simulink model "ClimaCenCfgMgr"  (R2022b)
settings: Solver=FixedStepDiscrete  StartTime=0.0  StopTime=10.0  FixedStep=0.1  RelTol=1e-3
(part 2 of 2)

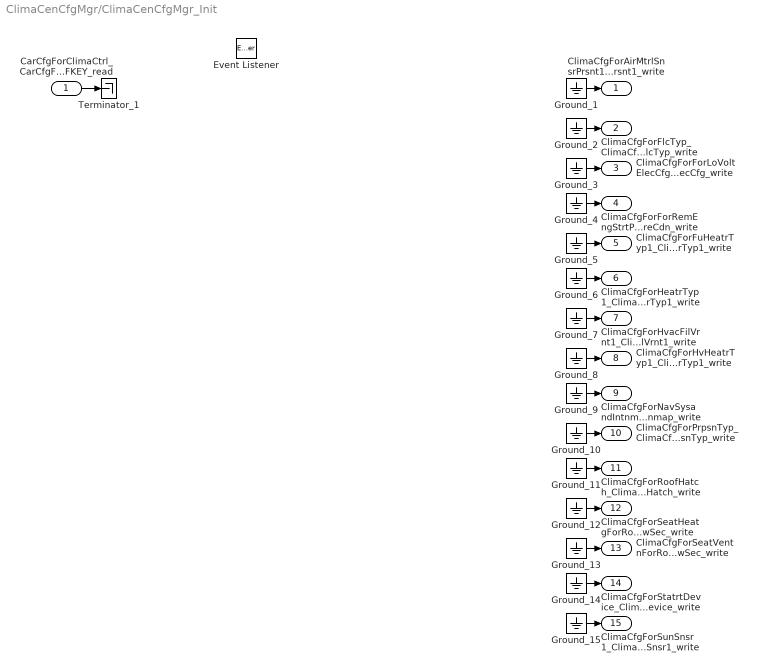
== system ClimaCenCfgMgr/ClimaCenCfgMgr_Init ==
  CarCfgForClimaCtrl_CarCfgForRFKEY_read : Inport
  Event Listener : EventListener  EventName=reset
  Ground_{1..15} (15 alike) : Ground
  Terminator_1 : Terminator
  ClimaCfgForAirMtrlSnsrPrsnt1_ClimaCfgForAirMtrlSnsrPrsnt1_write : Outport
  ClimaCfgForFlcTyp_ClimaCfgForFlcTyp_write : Outport  Port=2
  ClimaCfgForForLoVoltElecCfg_ClimaCfgForForLoVoltElecCfg_write : Outport  Port=3
  ClimaCfgForForRemEngStrtPreCdn_ClimaCfgForForRemEngStrtPreCdn_write : Outport  Port=4
  ClimaCfgForFuHeatrTyp1_ClimaCfgForFuHeatrTyp1_write : Outport  Port=5
  ClimaCfgForHeatrTyp1_ClimaCfgForHeatrTyp1_write : Outport  Port=6
  ClimaCfgForHvacFilVrnt1_ClimaCfgForHvacFilVrnt1_write : Outport  Port=7
  ClimaCfgForHvHeatrTyp1_ClimaCfgForHvHeatrTyp1_write : Outport  Port=8
  ClimaCfgForNavSysandIntnmap_ClimaCfgForNavSysandIntnmap_write : Outport  Port=9
  ClimaCfgForPrpsnTyp_ClimaCfgForPrpsnTyp_write : Outport  Port=10
  ClimaCfgForRoofHatch_ClimaCfgForRoofHatch_write : Outport  Port=11
  ClimaCfgForSeatHeatgForRowSec_ClimaCfgForSeatHeatgForRowSec_write : Outport  Port=12
  ClimaCfgForSeatVentnForRowSec_ClimaCfgForSeatVentnForRowSec_write : Outport  Port=13
  ClimaCfgForStatrtDevice_ClimaCfgForStartDevice_write : Outport  Port=14
  ClimaCfgForSunSnsr1_ClimaCfgForSunSnsr1_write : Outport  Port=15
  wires:
  CarCfgForClimaCtrl_CarCfgForRFKEY_read/1 -> Terminator_1/1
  Ground_1/1 -> ClimaCfgForAirMtrlSnsrPrsnt1_ClimaCfgForAirMtrlSnsrPrsnt1_write/1
  Ground_2/1 -> ClimaCfgForFlcTyp_ClimaCfgForFlcTyp_write/1
  Ground_3/1 -> ClimaCfgForForLoVoltElecCfg_ClimaCfgForForLoVoltElecCfg_write/1
  Ground_4/1 -> ClimaCfgForForRemEngStrtPreCdn_ClimaCfgForForRemEngStrtPreCdn_write/1
  Ground_5/1 -> ClimaCfgForFuHeatrTyp1_ClimaCfgForFuHeatrTyp1_write/1
  Ground_6/1 -> ClimaCfgForHeatrTyp1_ClimaCfgForHeatrTyp1_write/1
  Ground_7/1 -> ClimaCfgForHvacFilVrnt1_ClimaCfgForHvacFilVrnt1_write/1
  Ground_8/1 -> ClimaCfgForHvHeatrTyp1_ClimaCfgForHvHeatrTyp1_write/1
  Ground_9/1 -> ClimaCfgForNavSysandIntnmap_ClimaCfgForNavSysandIntnmap_write/1
  Ground_10/1 -> ClimaCfgForPrpsnTyp_ClimaCfgForPrpsnTyp_write/1
  Ground_11/1 -> ClimaCfgForRoofHatch_ClimaCfgForRoofHatch_write/1
  Ground_12/1 -> ClimaCfgForSeatHeatgForRowSec_ClimaCfgForSeatHeatgForRowSec_write/1
  Ground_13/1 -> ClimaCfgForSeatVentnForRowSec_ClimaCfgForSeatVentnForRowSec_write/1
  Ground_14/1 -> ClimaCfgForStatrtDevice_ClimaCfgForStartDevice_write/1
  Ground_15/1 -> ClimaCfgForSunSnsr1_ClimaCfgForSunSnsr1_write/1

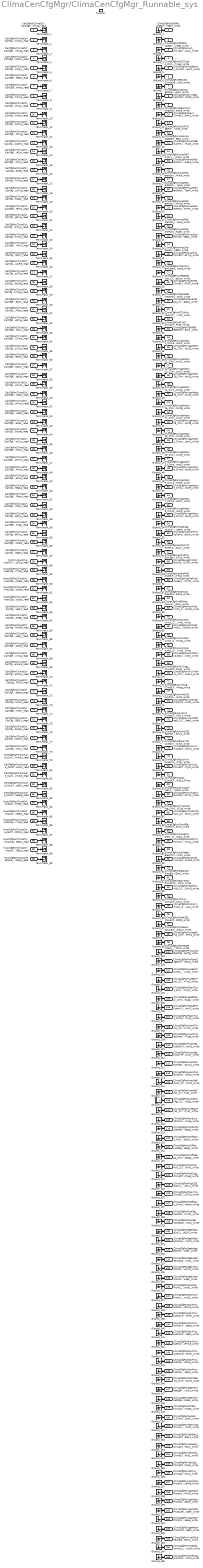
== system ClimaCenCfgMgr/ClimaCenCfgMgr_Runnable_sys ==
  CarCfgForClimaCtrl_CarCfgForAirClng_read : Inport
  CarCfgForClimaCtrl_CarCfgForAirClnrAdv_read : Inport  Port=2
  CarCfgForClimaCtrl_CarCfgForAirFrag_read : Inport  Port=3
  CarCfgForClimaCtrl_CarCfgForAirMtrlSnsr_read : Inport  Port=4
  CarCfgForClimaCtrl_CarCfgForAirQly_read : Inport  Port=5
  CarCfgForClimaCtrl_CarCfgForAmntOfSeat_read : Inport  Port=6
  CarCfgForClimaCtrl_CarCfgForAutDemst_read : Inport  Port=7
  CarCfgForClimaCtrl_CarCfgForAuxWtrPmp_read : Inport  Port=8
  CarCfgForClimaCtrl_CarCfgForBypVlvInHvac_read : Inport  Port=9
  CarCfgForClimaCtrl_CarCfgForClsVlvInHvac_read : Inport  Port=10
  CarCfgForClimaCtrl_CarCfgForCmprAcTyp_read : Inport  Port=11
  CarCfgForClimaCtrl_CarCfgForCmptmtClng_read : Inport  Port=12
  CarCfgForClimaCtrl_CarCfgForElecVentAdjmt_read : Inport  Port=13
  CarCfgForClimaCtrl_CarCfgForExtVentnClimaCtrl_read : Inport  Port=14
  CarCfgForClimaCtrl_CarCfgForFlcTyp_read : Inport  Port=15
  CarCfgForClimaCtrl_CarCfgForFoldCSD_read : Inport  Port=16
  CarCfgForClimaCtrl_CarCfgForFuTyp_read : Inport  Port=17
  CarCfgForClimaCtrl_CarCfgForGlassTyp_read : Inport  Port=18
  CarCfgForClimaCtrl_CarCfgForHeadRadar_read : Inport  Port=19
  CarCfgForClimaCtrl_CarCfgForHeatPmp_read : Inport  Port=20
  CarCfgForClimaCtrl_CarCfgForHeatrDurgDrvg_read : Inport  Port=21
  CarCfgForClimaCtrl_CarCfgForHeatrTyp_read : Inport  Port=22
  CarCfgForClimaCtrl_CarCfgForHvacFilTyp_read : Inport  Port=23
  CarCfgForClimaCtrl_CarCfgForHvacFlrSnsrForT_read : Inport  Port=24
  CarCfgForClimaCtrl_CarCfgForHvacHeatrSnsrT_read : Inport  Port=25
  CarCfgForClimaCtrl_CarCfgForHvacRe_read : Inport  Port=26
  CarCfgForClimaCtrl_CarCfgForHvacTyp_read : Inport  Port=27
  CarCfgForClimaCtrl_CarCfgForLoVoltElecCfg_read : Inport  Port=28
  CarCfgForClimaCtrl_CarCfgForNavSysandIntnmap_read : Inport  Port=29
  CarCfgForClimaCtrl_CarCfgForNrOfDoor_read : Inport  Port=30
  CarCfgForClimaCtrl_CarCfgForPipeInFuTank_read : Inport  Port=31
  CarCfgForClimaCtrl_CarCfgForPreClng_read : Inport  Port=32
  CarCfgForClimaCtrl_CarCfgForPrkgCDSNotAvl_read : Inport  Port=33
  CarCfgForClimaCtrl_CarCfgForPrkgClima_read : Inport  Port=34
  CarCfgForClimaCtrl_CarCfgForPrpsnTyp_read : Inport  Port=35
  CarCfgForClimaCtrl_CarCfgForRemEngStrtPreCdn_read : Inport  Port=36
  CarCfgForClimaCtrl_CarCfgForRoofHatch_read : Inport  Port=37
  CarCfgForClimaCtrl_CarCfgForSeatHeatgCal_read : Inport  Port=38
  CarCfgForClimaCtrl_CarCfgForSeatHeatgForRowFirst_read : Inport  Port=39
  CarCfgForClimaCtrl_CarCfgForSeatHeatgForRowSec_read : Inport  Port=40
  CarCfgForClimaCtrl_CarCfgForSeatVentnForRowFirst_read : Inport  Port=41
  CarCfgForClimaCtrl_CarCfgForSeatVentnForRowSec_read : Inport  Port=42
  CarCfgForClimaCtrl_CarCfgForSecRowVentnCenter_read : Inport  Port=43
  CarCfgForClimaCtrl_CarCfgForSecRowVentnSide_read : Inport  Port=44
  CarCfgForClimaCtrl_CarCfgForSmallFlowCyc_read : Inport  Port=45
  CarCfgForClimaCtrl_CarCfgForSmkSnsr_read : Inport  Port=46
  CarCfgForClimaCtrl_CarCfgForSnsrForCmptmtT_read : Inport  Port=47
  CarCfgForClimaCtrl_CarCfgForSnsrHumPrsnt_read : Inport  Port=48
  CarCfgForClimaCtrl_CarCfgForStartDevice_read : Inport  Port=49
  CarCfgForClimaCtrl_CarCfgForSteerWhlHeatg_read : Inport  Port=50
  CarCfgForClimaCtrl_CarCfgForSteerWhlPosn_read : Inport  Port=51
  CarCfgForClimaCtrl_CarCfgForSunSnsr_read : Inport  Port=52
  CarCfgForClimaCtrl_CarCfgForTelmNodTyp_read : Inport  Port=53
  CarCfgForClimaCtrl_CarCfgForThermMngt_read : Inport  Port=54
  CarCfgForClimaCtrl_CarCfgForVehTyp_read : Inport  Port=55
  CarCfgForClimaCtrl_CarCfgForVgaMotorExistSts_read : Inport  Port=56
  CarCfgForClimaCtrl_CarCfgForWindHeatd_read : Inport  Port=57
  CooltVlvOutpTyp_CooltVlvOutpTyp_read : Inport  Port=58
  HvacCfgForClimaCtrl_HvacCfgForHvacFrontBlowerType_read : Inport  Port=59
  HvacCfgForClimaCtrl_HvacCfgForHvacMiscExistSts_read : Inport  Port=60
  HvacCfgForClimaCtrl_HvacCfgForHvacMotorExistSts_read : Inport  Port=61
  HvacCfgForClimaCtrl_HvacCfgForHvacSensorExistSts_read : Inport  Port=62
  CarCfgForClimaCtrl_CarCfgFor3RDROWSEATHEAT_read : Inport  Port=63
  CarCfgForClimaCtrl_CarCfgFor3RDROWSEATVENTILATED_read : Inport  Port=64
  CarCfgForClimaCtrl_CarCfgForBRAND_read : Inport  Port=65
  CarCfgForClimaCtrl_CarCfgForCabinOxgenSupply_read : Inport  Port=66
  CarCfgForClimaCtrl_CarCfgForElectricLiftingModule_read : Inport  Port=67
  CarCfgForClimaCtrl_CarCfgForFloatingDriving_read : Inport  Port=68
  CarCfgForClimaCtrl_CarCfgForInteriorViewCamera_read : Inport  Port=69
  CarCfgForClimaCtrl_CarCfgForLifeDetection_read : Inport  Port=70
  CarCfgForClimaCtrl_CarCfgForMARKET_read : Inport  Port=71
  CarCfgForClimaCtrl_CarCfgForPowerSeats_read : Inport  Port=72
  CarCfgForClimaCtrl_CarCfgForPTCInFrontHVAC_read : Inport  Port=73
  CarCfgForClimaCtrl_CarCfgForRFKEY_read : Inport  Port=74
  CarCfgForClimaCtrl_CarCfgForTEMPEREDSTORAGEBOX_read : Inport  Port=75
  CarCfgForClimaCtrl_CarCfgForVentLiftMotorExiSts_read : Inport  Port=76
  CarCfgForClimaCtrl_CarCfgForWadingAssistSystem_read : Inport  Port=77
  CarCfgForClimaCtrl_CarCfgWindFollowHumanMov_read : Inport  Port=78
  CenCfgMgrForCompile_CarCfgForAirClnrAdv_read : Inport  Port=79
  CenCfgMgrForCompile_CarCfgForAutDemst_read : Inport  Port=80
  CenCfgMgrForCompile_CarCfgForCmprAcTyp3_read : Inport  Port=81
  CenCfgMgrForCompile_CarCfgForHeatrTypGly_read : Inport  Port=82
  CenCfgMgrForCompile_CarCfgForSteerWhlHeatg_read : Inport  Port=83
  HvacCfgForClimaCtrl_HvacCfgForAQSTypes_read : Inport  Port=84
  HvacCfgForClimaCtrl_HvacCfgForHvacLowSideRETXVESts_read : Inport  Port=85
  HvacCfgForClimaCtrl_HvacCfgForHvacReBlowerType_read : Inport  Port=86
  HvacCfgForClimaCtrl_HvacCfgForHvacReVentPstn_read : Inport  Port=87
  HvacCfgForClimaCtrl_HvacCfgForHvacSnsrRCmptmtESts_read : Inport  Port=88
  HvacCfgForClimaCtrl_HvacCfgForHvacSnsrReHexESts_read : Inport  Port=89
  HvacCfgReqVersionReplace_HvacCfgReqVersionReplace_read : Inport  Port=90
  function : TriggerPort  TriggerType=function-call  VariantControl=(inherit)  SampleTimeType=periodic  SampleTime=-1
  Ground_{1..115,117..165} (164 alike) : Ground
  Ground_116 : Subsystem
  Terminator_{1..90} (90 alike) : Terminator
  ClimaCfgFor3RDROWSEATHEAT_ClimaCfgFor3RDROWSEATHEAT_write : Outport
      settings: EnsureOutportIsVirtual=on
  ClimaCfgFor3RDROWSEATVENTILATED_ClimaCfgFor3RDROWSEATVENTILATED_write : Outport  Port=2
      settings: EnsureOutportIsVirtual=on
  ClimaCfgForAAC_ClimaCfgForAAC_write : Outport  Port=3
      settings: EnsureOutportIsVirtual=on
  ClimaCfgForAQSTypes_ClimaCfgForAQSTypes_write : Outport  Port=4
      settings: EnsureOutportIsVirtual=on
  ClimaCfgForAutDemst_ClimaCfgForAutDemst_write : Outport  Port=5
      settings: EnsureOutportIsVirtual=on
  ClimaCfgForBRAND_ClimaCfgForBRAND_write : Outport  Port=6
      settings: EnsureOutportIsVirtual=on
  ClimaCfgForCabinOxgenSupply_ClimaCfgForCabinOxgenSupply_write : Outport  Port=7
      settings: EnsureOutportIsVirtual=on
  ClimaCfgForDrvrSeatVentWthSmf2_ClimaCfgForDrvrSeatVentWthSmf2_write : Outport  Port=8
      settings: EnsureOutportIsVirtual=on
  ClimaCfgForElectricLiftingModule_ClimaCfgForElectricLiftingModule_write : Outport  Port=9
      settings: EnsureOutportIsVirtual=on
  ClimaCfgForErev1_ClimaCfgForErev1_write : Outport  Port=10
      settings: EnsureOutportIsVirtual=on
  ClimaCfgForFLOATINGDRVING_ClimaCfgForFLOATINGDRVING_write : Outport  Port=11
      settings: EnsureOutportIsVirtual=on
  ClimaCfgForHvacLowSideRETXVESts_ClimaCfgForHvacLowSideRETXVESts_write : Outport  Port=12
      settings: EnsureOutportIsVirtual=on
  ClimaCfgForHvacReBlowerType_ClimaCfgForHvacReBlowerType_write : Outport  Port=13
      settings: EnsureOutportIsVirtual=on
  ClimaCfgForHvacReTmpZn_ClimaCfgForHvacReTmpZn_write : Outport  Port=14
      settings: EnsureOutportIsVirtual=on
  ClimaCfgForHvacReVentPstn_ClimaCfgForRowSecAtFlrLeRe_write : Outport  Port=15
      settings: EnsureOutportIsVirtual=on
  ClimaCfgForHvacReVentPstn_ClimaCfgForRowSecAtFlrRiRe_write : Outport  Port=16
      settings: EnsureOutportIsVirtual=on
  ClimaCfgForHvacReVentPstn_ClimaCfgForRowSecAtVentSideTopLe_write : Outport  Port=17
      settings: EnsureOutportIsVirtual=on
  ClimaCfgForHvacReVentPstn_ClimaCfgForRowSecAtVentSideTopRi_write : Outport  Port=18
      settings: EnsureOutportIsVirtual=on
  ClimaCfgForHvacReVentPstn_ClimaCfgForRowThrdAtFlrLe_write : Outport  Port=19
      settings: EnsureOutportIsVirtual=on
  ClimaCfgForHvacReVentPstn_ClimaCfgForRowThrdAtFlrRi_write : Outport  Port=20
      settings: EnsureOutportIsVirtual=on
  ClimaCfgForHvacReVentPstn_ClimaCfgForRowThrdAtVentSideTopLe_write : Outport  Port=21
      settings: EnsureOutportIsVirtual=on
  ClimaCfgForHvacReVentPstn_ClimaCfgForRowThrdAtVentSideTopRi_write : Outport  Port=22
      settings: EnsureOutportIsVirtual=on
  ClimaCfgForHvacSnsrRCmptmtESts_ClimaCfgForHvacSnsrRCmptmtESts_write : Outport  Port=23
      settings: EnsureOutportIsVirtual=on
  ClimaCfgForHvacSnsrReHexESts_ClimaCfgForHvacSnsrReHexESts_write : Outport  Port=24
      settings: EnsureOutportIsVirtual=on
  ClimaCfgForHvacTyp_ClimaCfgForHvacTyp_write : Outport  Port=25
      settings: EnsureOutportIsVirtual=on
  ClimaCfgForInteriorViewCamera_ClimaCfgForInteriorViewCamera_write : Outport  Port=26
      settings: EnsureOutportIsVirtual=on
  ClimaCfgForLifeDetection_ClimaCfgForLifeDetection_write : Outport  Port=27
      settings: EnsureOutportIsVirtual=on
  ClimaCfgForMARKET_ClimaCfgForMARKET_write : Outport  Port=28
      settings: EnsureOutportIsVirtual=on
  ClimaCfgForPassSeatVentWthSmf2_ClimaCfgForPassSeatVentWthSmf2_write : Outport  Port=29
      settings: EnsureOutportIsVirtual=on
  ClimaCfgForPowerSeats_ClimaCfgForPowerSeats_write : Outport  Port=30
      settings: EnsureOutportIsVirtual=on
  ClimaCfgForPTCInFrontHVAC_ClimaCfgForPTCInFrontHVAC_write : Outport  Port=31
      settings: EnsureOutportIsVirtual=on
  ClimaCfgForRFKEY_ClimaCfgForRFKEY_write : Outport  Port=32
      settings: EnsureOutportIsVirtual=on
  ClimaCfgForTEMPEREDSTORAGEBOX_ClimaCfgForTEMPEREDSTORAGEBOX_write : Outport  Port=33
      settings: EnsureOutportIsVirtual=on
  ClimaCfgForVgaMotor1_ClimaCfgForVgaMotor1_write : Outport  Port=34
      settings: EnsureOutportIsVirtual=on
  ClimaCfgForVgaMotor10_ClimaCfgForVgaMotor10_write : Outport  Port=35
      settings: EnsureOutportIsVirtual=on
  ClimaCfgForVgaMotor11_ClimaCfgForVgaMotor11_write : Outport  Port=36
      settings: EnsureOutportIsVirtual=on
  ClimaCfgForVgaMotor12_ClimaCfgForVgaMotor12_write : Outport  Port=37
      settings: EnsureOutportIsVirtual=on
  ClimaCfgForVgaMotor13_ClimaCfgForVgaMotor13_write : Outport  Port=38
      settings: EnsureOutportIsVirtual=on
  ClimaCfgForVgaMotor14_ClimaCfgForVgaMotor14_write : Outport  Port=39
      settings: EnsureOutportIsVirtual=on
  ClimaCfgForVgaMotor15_ClimaCfgForVgaMotor15_write : Outport  Port=40
      settings: EnsureOutportIsVirtual=on
  ClimaCfgForVgaMotor16_ClimaCfgForVgaMotor16_write : Outport  Port=41
      settings: EnsureOutportIsVirtual=on
  ClimaCfgForVgaMotor17_ClimaCfgForVgaMotor17_write : Outport  Port=42
      settings: EnsureOutportIsVirtual=on
  ClimaCfgForVgaMotor18_ClimaCfgForVgaMotor18_write : Outport  Port=43
      settings: EnsureOutportIsVirtual=on
  ClimaCfgForVgaMotor19_ClimaCfgForVgaMotor19_write : Outport  Port=44
      settings: EnsureOutportIsVirtual=on
  ClimaCfgForVgaMotor2_ClimaCfgForVgaMotor2_write : Outport  Port=45
      settings: EnsureOutportIsVirtual=on
  ClimaCfgForVgaMotor20_ClimaCfgForVgaMotor20_write : Outport  Port=46
      settings: EnsureOutportIsVirtual=on
  ClimaCfgForVgaMotor3_ClimaCfgForVgaMotor3_write : Outport  Port=47
      settings: EnsureOutportIsVirtual=on
  ClimaCfgForVgaMotor4_ClimaCfgForVgaMotor4_write : Outport  Port=48
      settings: EnsureOutportIsVirtual=on
  ClimaCfgForVgaMotor5_ClimaCfgForVgaMotor5_write : Outport  Port=49
      settings: EnsureOutportIsVirtual=on
  ClimaCfgForVgaMotor6_ClimaCfgForVgaMotor6_write : Outport  Port=50
      settings: EnsureOutportIsVirtual=on
  ClimaCfgForVgaMotor7_ClimaCfgForVgaMotor7_write : Outport  Port=51
      settings: EnsureOutportIsVirtual=on
  ClimaCfgForVgaMotor8_ClimaCfgForVgaMotor8_write : Outport  Port=52
      settings: EnsureOutportIsVirtual=on
  ClimaCfgForVgaMotor9_ClimaCfgForVgaMotor9_write : Outport  Port=53
      settings: EnsureOutportIsVirtual=on
  ClimaCfgForWadingAssistSystem_ClimaCfgForWadingAssistSystem_write : Outport  Port=54
      settings: EnsureOutportIsVirtual=on
  ClimaCfgHvacForFrntLeTempActr_ClimaCfgHvacForFrntLeTempActr_write : Outport  Port=55
      settings: EnsureOutportIsVirtual=on
  ClimaCfgHvacForFrntRiTempActr_ClimaCfgHvacForFrntRiTempActr_write : Outport  Port=56
      settings: EnsureOutportIsVirtual=on
  ClimaCfgHvacFrntForRowSecForActrTLe_ClimaCfgHvacFrntForRowSecForActrTLe_write : Outport  Port=57
      settings: EnsureOutportIsVirtual=on
  ClimaCfgHvacFrntForRowSecForActrTRi_ClimaCfgHvacFrntForRowSecForActrTRi_write : Outport  Port=58
      settings: EnsureOutportIsVirtual=on
  ClimaCfgHvacReForRowSecForActrTLe_ClimaCfgHvacReForRowSecForActrTLe_write : Outport  Port=59
      settings: EnsureOutportIsVirtual=on
  ClimaCfgHvacReForRowSecForActrTRi_ClimaCfgHvacReForRowSecForActrTRi_write : Outport  Port=60
      settings: EnsureOutportIsVirtual=on
  ClimaCfgReqVersionReplace_ClimaCfgReqVersionReplace_write : Outport  Port=61
      settings: EnsureOutportIsVirtual=on
  ClimaCfgWindFollowHumanMov_ClimaCfgWindFollowHumanMov_write : Outport  Port=62
      settings: EnsureOutportIsVirtual=on
  ClimaCfgForActrModVrnt1_ClimaCfgForActrInd1_write : Outport  Port=63
      settings: EnsureOutportIsVirtual=on
  ClimaCfgForActrModVrnt1_ClimaCfgForActrModVrnt1_write : Outport  Port=64
      settings: EnsureOutportIsVirtual=on
  ClimaCfgForActrModVrnt1_ClimaCfgForActrModVrntCen_write : Outport  Port=65
      settings: EnsureOutportIsVirtual=on
  ClimaCfgForActrModVrnt2_ClimaCfgForActrInd2_write : Outport  Port=66
      settings: EnsureOutportIsVirtual=on
  ClimaCfgForActrModVrnt2_ClimaCfgForActrModVrnt2_write : Outport  Port=67
      settings: EnsureOutportIsVirtual=on
  ClimaCfgForActrRecircAndOsaCmnPrsnt1_ClimaCfgForActrRecircAndOsaCmnPrsnt1_write : Outport  Port=68
      settings: EnsureOutportIsVirtual=on
  ClimaCfgForAirClng1_ClimaCfgForAirClng1_write : Outport  Port=69
      settings: EnsureOutportIsVirtual=on
  ClimaCfgForAirClnrAdv1_ClimaCfgForAirClnrAdv1_write : Outport  Port=70
      settings: EnsureOutportIsVirtual=on
  ClimaCfgForAirFrag_ClimaCfgForAirFrag_write : Outport  Port=71
      settings: EnsureOutportIsVirtual=on
  ClimaCfgForAirMtrlSnsrAPMAPrsnt1_ClimaCfgForAirMtrlSnsrAPMAPrsnt1_write : Outport  Port=72
      settings: EnsureOutportIsVirtual=on
  ClimaCfgForAirMtrlSnsrPrsnt1_ClimaCfgForAirMtrlSnsrPrsnt1_write : Outport  Port=73
      settings: EnsureOutportIsVirtual=on
  ClimaCfgForAirQly1_ClimaCfgForAirQly1_write : Outport  Port=74
      settings: EnsureOutportIsVirtual=on
  ClimaCfgForAmntOfSeat1_ClimaCfgForAmntOfSeat1_write : Outport  Port=75
      settings: EnsureOutportIsVirtual=on
  ClimaCfgForAmntOfSeatRow1_ClimaCfgForAmntOfSeatRow1_write : Outport  Port=76
      settings: EnsureOutportIsVirtual=on
  ClimaCfgForBlowrInRowSecVentSidePrsnt1_ClimaCfgForBlowrInRowSecVentSidePrsnt1_write : Outport  Port=77
      settings: EnsureOutportIsVirtual=on
  ClimaCfgForBypVlvInHvacExtCtrl1_ClimaCfgForBypVlvInHvacExtCtrl1_write : Outport  Port=78
      settings: EnsureOutportIsVirtual=on
  ClimaCfgForBypVlvInHvacPrsnt1_ClimaCfgForBypVlvInHvacPrsnt1_write : Outport  Port=79
      settings: EnsureOutportIsVirtual=on
  ClimaCfgForCarTyp1_ClimaCfgForCarTyp1_write : Outport  Port=80
      settings: EnsureOutportIsVirtual=on
  ClimaCfgForCds1_ClimaCfgForCds1_write : Outport  Port=81
      settings: EnsureOutportIsVirtual=on
  ClimaCfgForClimaCtrlMan1_ClimaCfgForClimaCtrlMan1_write : Outport  Port=82
      settings: EnsureOutportIsVirtual=on
  ClimaCfgForClimaCtrlSwtModlPrsnt_ClimaCfgForClimaCtrlSwtModlPrsnt_write : Outport  Port=83
      settings: EnsureOutportIsVirtual=on
  ClimaCfgForCmptmtClng_ClimaCfgForCmptmtClng_write : Outport  Port=84
      settings: EnsureOutportIsVirtual=on
  ClimaCfgForCmptmtSnsr1_ClimaCfgForCmptmtSnsr1_write : Outport  Port=85
      settings: EnsureOutportIsVirtual=on
  ClimaCfgForComIfGenn2ForTelm1_ClimaCfgForComIfGenn2ForTelm1_write : Outport  Port=86
      settings: EnsureOutportIsVirtual=on
  ClimaCfgForCoolgPreCdn1_ClimaCfgForCoolgPreCdn1_write : Outport  Port=87
      settings: EnsureOutportIsVirtual=on
  ClimaCfgForCooltCircForClima1_ClimaCfgForCooltCircForClima1_write : Outport  Port=88
      settings: EnsureOutportIsVirtual=on
  ClimaCfgForCpilHeatrElecPrsnt1_ClimaCfgForCpilHeatrElecPrsnt1_write : Outport  Port=89
      settings: EnsureOutportIsVirtual=on
  ClimaCfgForEcuCooltVlvOutp1_ClimaCfgForEcuCooltVlvOutp1_write : Outport  Port=90
      settings: EnsureOutportIsVirtual=on
  ClimaCfgForEngCooltPmpElec1_ClimaCfgForEngCooltPmpElec1_write : Outport  Port=91
      settings: EnsureOutportIsVirtual=on
  ClimaCfgForExtVentnClimaCtrl_ClimaCfgForExtVentnClimaCtrl_write : Outport  Port=92
      settings: EnsureOutportIsVirtual=on
  ClimaCfgForFanPotiCtrld1_ClimaCfgForFanPotiCtrld1_write : Outport  Port=93
      settings: EnsureOutportIsVirtual=on
  ClimaCfgForFlcTyp_ClimaCfgForFlcTyp_write : Outport  Port=94
      settings: EnsureOutportIsVirtual=on
  ClimaCfgForFlrSnsrInHvac1_ClimaCfgForFlrSnsrInHvac1_write : Outport  Port=95
      settings: EnsureOutportIsVirtual=on
  ClimaCfgForFoldCSD_ClimaCfgForFoldCSD_write : Outport  Port=96
      settings: EnsureOutportIsVirtual=on
  ClimaCfgForForElecVentAdjmt_ClimaCfgForForElecVentAdjmt_write : Outport  Port=97
      settings: EnsureOutportIsVirtual=on
  ClimaCfgForForHeatPmp_ClimaCfgForForHeatPmp_write : Outport  Port=98
      settings: EnsureOutportIsVirtual=on
  ClimaCfgForForHeatPmpGly_ClimaCfgForForHeatPmp_write : Outport  Port=99
      settings: EnsureOutportIsVirtual=on
  ClimaCfgForForLoVoltElecCfg_ClimaCfgForForLoVoltElecCfg_write : Outport  Port=100
      settings: EnsureOutportIsVirtual=on
  ClimaCfgForForRemEngStrtPreCdn_ClimaCfgForForRemEngStrtPreCdn_write : Outport  Port=101
      settings: EnsureOutportIsVirtual=on
  ClimaCfgForFuHeatrFuLine1_ClimaCfgForFuHeatrFuLine1_write : Outport  Port=102
      settings: EnsureOutportIsVirtual=on
  ClimaCfgForFuHeatrTyp1_ClimaCfgForFuHeatrTyp1_write : Outport  Port=103
      settings: EnsureOutportIsVirtual=on
  ClimaCfgForGlassTyp1_ClimaCfgForGlassTyp1_write : Outport  Port=104
      settings: EnsureOutportIsVirtual=on
  ClimaCfgForHeadRadar_ClimaCfgForHeadRadar_write : Outport  Port=105
      settings: EnsureOutportIsVirtual=on
  ClimaCfgForHeatgPreCdnAvl1_ClimaCfgForHeatgPreCdnAvl1_write : Outport  Port=106
      settings: EnsureOutportIsVirtual=on
  ClimaCfgForHeatrTyp1_ClimaCfgForHeatrTyp1_write : Outport  Port=107
      settings: EnsureOutportIsVirtual=on
  ClimaCfgForHvacFilVrnt1_ClimaCfgForHvacFilVrnt1_write : Outport  Port=108
      settings: EnsureOutportIsVirtual=on
  ClimaCfgForHvacFrontBlowerType_ClimaCfgForHvacFrontBlowerType_write : Outport  Port=109
      settings: EnsureOutportIsVirtual=on
  ClimaCfgForHvacHeatrElecFrntPrsnt1_ClimaCfgForHvacHeatrElecFrntPrsnt1_write : Outport  Port=110
      settings: EnsureOutportIsVirtual=on
  ClimaCfgForHvacHeatrSnsrTPrsnt1_ClimaCfgForHvacHeatrSnsrTPrsnt1_write : Outport  Port=111
      settings: EnsureOutportIsVirtual=on
  ClimaCfgForHvacManWithPartRecirc2_ClimaCfgForHvacManWithPartRecirc2_write : Outport  Port=112
      settings: EnsureOutportIsVirtual=on
  ClimaCfgForHvacMiscExistSts_ClimaCfgForHvacMiscExistSts_write : Outport  Port=113
      settings: EnsureOutportIsVirtual=on
  ClimaCfgForHvacRePrsnt1_ClimaCfgForHvacRePrsnt1_write : Outport  Port=114
      settings: EnsureOutportIsVirtual=on
  ClimaCfgForHvacReTyp1_ClimaCfgForHvacReTyp1_write : Outport  Port=115
      settings: EnsureOutportIsVirtual=on
  ClimaCfgForHvacSnsrCfg1_ClimaCfgForHvacSnsrCfg1_write : Outport  Port=116
      settings: EnsureOutportIsVirtual=on
  ClimaCfgForHvHeatrTyp1_ClimaCfgForHvHeatrTyp1_write : Outport  Port=117
      settings: EnsureOutportIsVirtual=on
  ClimaCfgForNavSysandIntnmap_ClimaCfgForNavSysandIntnmap_write : Outport  Port=118
      settings: EnsureOutportIsVirtual=on
  ClimaCfgForNrOfAirDistbnSetg1_ClimaCfgForNrOfAirDistbnSetg1_write : Outport  Port=119
      settings: EnsureOutportIsVirtual=on
  ClimaCfgForNrOfDoor1_ClimaCfgForNrOfDoor1_write : Outport  Port=120
      settings: EnsureOutportIsVirtual=on
  ClimaCfgForNrOfFanLvlSetg1_ClimaCfgForNrOfFanLvlSetg1_write : Outport  Port=121
      settings: EnsureOutportIsVirtual=on
  ClimaCfgForNrOfTSetg1_ClimaCfgForNrOfTSetg1_write : Outport  Port=122